simulink model "wind1000_BUS_Original"  (R2024a)
settings: Solver=ode45  StartTime=parSimulacio.ti  StopTime=40  MaxStep=parSimulacio.pas  RelTol=1e-6  AbsTol=1e-6  EnableMultiTasking=on
model workspace (MAT data in the model file):
  tt_vw = Simulink.Parameter
  tt_vw.Complexity = 'real'
  tt_vw.Dimensions = [0 0]
  tt_vw.DataType = 'auto'
values from neighbouring files (symbols this model uses):
  L = L'    [parameters_windFarm2.m]
  nu = size(S, 1)    [parameters_windFarm2.m]
undefined symbols (not defined in the file or in its folder): parControl, parConvertidor, parGenerador, parGrid, parMecanica, parSimulacio, S, w0, windspeed
(part 1 of 218)
settings Model: SimulationMode=accelerator  Creator=adria  LastModifiedBy=hz1021
settings SolverCC: AlgebraicLoopSolver=TrustRegion
settings DataIOCC: LimitDataPoints=on  SaveFormat=Array  SignalLoggingSaveFormat=ModelDataLogs  SaveOutput=off  SaveTime=off  ReturnWorkspaceOutputs=off
settings OptimizationCC: ReuseModelBlockBuffer=off  InitFltsAndDblsToZero=on  LifeSpan=inf
settings DebuggingCC: SaveWithParameterizedLinksMsg=none  UnderspecifiedInitializationDetection=Classic  MergeDetectMultiDrivingBlocksExec=none  SignalResolutionControl=TryResolveAllWithWarning  SolverPrmCheckMsg=warning  FcnCallInpInsideContextMsg=warning  UnconnectedInputMsg=warning  UnconnectedOutputMsg=warning  UnconnectedLineMsg=warning  SFUnexpectedBacktrackingDiag=warning  SFNoUnconditionalDefaultTransitionDiag=warning  SFExecutionAtInitializationDiag=none  ArithmeticOperatorsInVariantConditions=warning
settings HardwareCC: ProdBitPerPointer=32  ProdBitPerSizeT=32  ProdBitPerPtrDiffT=32  ProdLargestAtomicFloat=None  ProdIntDivRoundTo=Undefined  ProdEndianess=Unspecified  ProdWordSize=32  ProdHWDeviceType=32-bit Generic
settings ModelReferenceCC: EnableRefExpFcnMdlSchedulingChecks=on  PropagateSignalLabelsOutOfModel=off
settings RTWCC: GPUComputeCapability=3.5  DLArmComputeVersion=19.05
settings CodeAppCC: StateflowObjectComments=on
settings GRTTargetCC: TargetFcnLib=ansi_tfl_tmw.mat  TargetLangStandard=C89/C90 (ANSI)  CombineOutputUpdateFcns=off  GRTInterface=on
settings ConfigComp: CovMetricSettings=dw  CovSaveCumulativeToWorkspaceVar=on  CovSaveSingleToWorkspaceVar=on

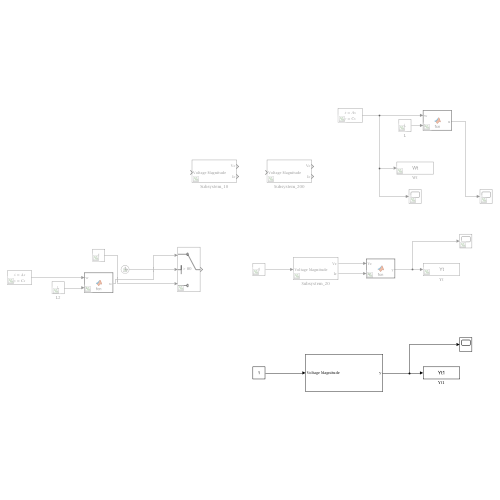
[thumbnail: Simulink's stored preview of the top-level diagram]
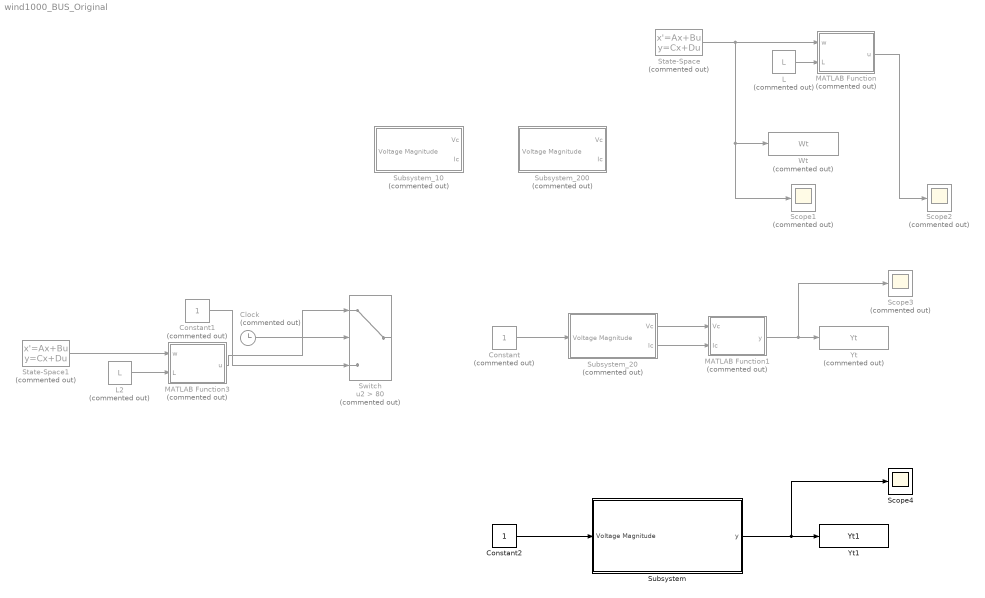
== system wind1000_BUS_Original ==
  Clock : Clock  Commented=on
  Constant : Constant  Commented=on
  Constant1 : Constant  Commented=on
  Constant2 : Constant
  L : Constant  Commented=on  Value=L
  L2 : Constant  Commented=on  Value=L
  MATLAB Function : MATLAB Function  Commented=on  TreatAsAtomicUnit=on
  MATLAB Function1 : MATLAB Function  Commented=on  TreatAsAtomicUnit=on
  MATLAB Function3 : MATLAB Function  Commented=on  TreatAsAtomicUnit=on
  Scope1 : Scope  Commented=on
  Scope2 : Scope  Commented=on
  Scope3 : Scope  Commented=on
  Scope4 : Scope
  State-Space : StateSpace  Commented=on  A=S  B=[]  C=eye(nu)  D=[]  InitialCondition=w0  AbsoluteTolerance=1e-6
  State-Space1 : StateSpace  Commented=on  A=S  B=[]  C=eye(nu)  D=[]  InitialCondition=w0  AbsoluteTolerance=1e-9
  Subsystem : Subsystem
  Subsystem_10 : Subsystem  Commented=on
  Subsystem_20 : Subsystem  Commented=on
  Subsystem_200 : Subsystem  Commented=on
  Switch : Switch  Commented=on  Criteria=u2 > Threshold  Threshold=80  SaturateOnIntegerOverflow=off  InputSameDT=off
  Wt : ToWorkspace  Commented=on  VariableName=Wt  SaveFormat=Timeseries  SampleTime=-1
  Yt : ToWorkspace  Commented=on  VariableName=Yt  SaveFormat=Timeseries  SampleTime=-1
  Yt1 : ToWorkspace  VariableName=Yt1  SaveFormat=Timeseries  SampleTime=-1
  wires:
  State-Space/1 -> Wt/1, Scope1/1, MATLAB Function/1(w)
  MATLAB Function1/1(y) -> Scope3/1, Yt/1
  MATLAB Function/1(u) -> Scope2/1
  L/1 -> MATLAB Function/2(L)
  Subsystem_20/1(Vc) -> MATLAB Function1/1(Vc)
  Subsystem_20/2(Ic) -> MATLAB Function1/2(Ic)
  Constant1/1 -> Switch/3
  L2/1 -> MATLAB Function3/2(L)
  Clock/1 -> Switch/2
  MATLAB Function3/1(u) -> Switch/1
  State-Space1/1 -> MATLAB Function3/1(w)
  Constant/1 -> Subsystem_20/1(Voltage Magnitude)
  Subsystem/1(y) -> Yt1/1, Scope4/1
  Constant2/1 -> Subsystem/1(Voltage Magnitude)

== system wind1000_BUS_Original/Subsystem ==
  Voltage Magnitude : Inport
  MATLAB Function2 : MATLAB Function  TreatAsAtomicUnit=on
  Subsystem_1 : Subsystem
  y : Outport
  wires:
  Subsystem_1/2(Ic) -> MATLAB Function2/2(Ic)
  Voltage Magnitude/1 -> Subsystem_1/1(Voltage Magnitude)
  Subsystem_1/1(Vc) -> MATLAB Function2/1(Vc)
  MATLAB Function2/1(y) -> y/1

== system wind1000_BUS_Original/Subsystem_10 ==
  Voltage Magnitude : Inport
  3phase2dq : Subsystem
  C at HV transf : Subsystem
  Gain1 : Gain  Gain=1/parGrid.WFtransf.rloss
  HV transf1 : Subsystem
  String1 : Subsystem
  Subtract1 : Sum  Inputs=+-
  Vc : Outport
  Ic : Outport  Port=2
  wires:
  Subtract1/1 -> C at HV transf/2(Itransf)
  3phase2dq/1(Vdq) -> HV transf1/1(Vin)
  HV transf1/1(Itransf) -> Subtract1/1
  C at HV transf/1(Vc) -> Vc/1, String1/1(V_in), HV transf1/2(Vc), Gain1/1
  Gain1/1 -> Subtract1/2
  Voltage Magnitude/1 -> 3phase2dq/1(Voltage Magnitude)
  String1/1(I_out) -> Ic/1, C at HV transf/1(Istrings)

== system wind1000_BUS_Original/Subsystem_20 ==
  Voltage Magnitude : Inport
  3phase2dq : Subsystem
  C at HV transf : Subsystem
  Gain1 : Gain  Gain=1/parGrid.WFtransf.rloss
  HV transf1 : Subsystem
  Scope1 : Scope
  Scope3 : Scope
  Subsystem : Subsystem
  Subtract1 : Sum  Inputs=+-
  Vc : Outport
  Ic : Outport  Port=2
  wires:
  Subtract1/1 -> Scope3/1, Ic/1, C at HV transf/2(Itransf)
  3phase2dq/1(Vdq) -> HV transf1/1(Vin)
  HV transf1/1(Itransf) -> Subtract1/1
  C at HV transf/1(Vc) -> Subsystem/1(V_in), Scope1/1, Vc/1, HV transf1/2(Vc), Gain1/1
  Gain1/1 -> Subtract1/2
  Voltage Magnitude/1 -> 3phase2dq/1(Voltage Magnitude)
  Subsystem/1(I_out_tol) -> C at HV transf/1(Istrings)

== system wind1000_BUS_Original/Subsystem_200 ==
  Voltage Magnitude : Inport
  3phase2dq : Subsystem
  C at HV transf : Subsystem
  Gain1 : Gain  Gain=1/parGrid.WFtransf.rloss
  HV transf1 : Subsystem
  Scope1 : Scope
  Scope3 : Scope
  Subsystem : Subsystem
  Subtract1 : Sum  Inputs=+-
  Vc : Outport
  Ic : Outport  Port=2
  wires:
  Subtract1/1 -> Scope3/1, Ic/1, C at HV transf/2(Itransf)
  3phase2dq/1(Vdq) -> HV transf1/1(Vin)
  HV transf1/1(Itransf) -> Subtract1/1
  C at HV transf/1(Vc) -> Subsystem/1(V_in), Scope1/1, Vc/1, HV transf1/2(Vc), Gain1/1
  Gain1/1 -> Subtract1/2
  Voltage Magnitude/1 -> 3phase2dq/1(Voltage Magnitude)
  Subsystem/1(I_out_tol) -> C at HV transf/1(Istrings)

== system wind1000_BUS_Original/Subsystem/Subsystem_1 ==
  Voltage Magnitude : Inport
  3phase2dq : Subsystem
  C at HV transf : Subsystem
  Gain1 : Gain  Gain=1/parGrid.WFtransf.rloss
  HV transf1 : Subsystem
  Scope1 : Scope
  Scope3 : Scope
  Subsystem : Subsystem
  Subtract1 : Sum  Inputs=+-
  Vc : Outport
  Ic : Outport  Port=2
  wires:
  Subtract1/1 -> Scope3/1, Ic/1, C at HV transf/2(Itransf)
  3phase2dq/1(Vdq) -> HV transf1/1(Vin)
  HV transf1/1(Itransf) -> Subtract1/1
  C at HV transf/1(Vc) -> Subsystem/1(V_in), Scope1/1, Vc/1, HV transf1/2(Vc), Gain1/1
  Gain1/1 -> Subtract1/2
  Voltage Magnitude/1 -> 3phase2dq/1(Voltage Magnitude)
  Subsystem/1(I_out_tol) -> C at HV transf/1(Istrings)

== system wind1000_BUS_Original/Subsystem_10/3phase2dq ==
  Voltage Magnitude : Inport
  Clock2 : Clock
  Font trifasica asimetrica1 : Subsystem  mask{tempPar=parGrid.WFtransf}
      mask prompts: tempPar "Estruc. Parametres"
      mask init:
      | we=tempPar.f*2*pi;
      | A_D=tempPar.V_D*sqrt(2)/sqrt(3);
      | A_I=tempPar.V_I*sqrt(2)/sqrt(3);
      | phi_D=tempPar.phi_D;
      | phi_I=tempPar.phi_I;
      | tt_D=tempPar.tt_D;
      | tt_I=tempPar.tt_I;
      | tA_D=tempPar.tA_D;
      | tA_I=tempPar.tA_I;
  Product : Product
  Vdq : Outport
  wires:
  Product/1 -> Vdq/1
  Clock2/1 -> Font trifasica asimetrica1/1(t)
  Font trifasica asimetrica1/1(y) -> Product/1
  Voltage Magnitude/1 -> Product/2

== system wind1000_BUS_Original/Subsystem_10/C at HV transf ==
  note: Vc_cable
  Istrings : Inport
  Itransf : Inport  Port=2
  Add : Sum  Inputs=+-
  produce Vc_cable : StateSpace  A=[0,-100*pi;100*pi,0]  B=eye(2)/parGrid.WFtransf.Cfilt  C=eye(2)  D=zeros(2,2)  InitialCondition=sqrt(2)*66000/sqrt(3)*[1;0]
  Vc : Outport
  wires:
  produce Vc_cable/1 -> Vc/1
  Add/1 -> produce Vc_cable/1
  Itransf/1 -> Add/2
  Istrings/1 -> Add/1

== system wind1000_BUS_Original/Subsystem_10/HV transf1 ==
  Vin : Inport
  Vc : Inport  Port=2
  1/L_Cable : Gain  Gain=1/parGrid.WFtransf.L
  A : Constant  Value=[parGrid.WFtransf.r,100*pi*parGrid.WFtransf.L;-100*pi*parGrid.WFtransf.L,parGrid.WFtransf.r]
  Add : Sum  Inputs=+--
  Integrator : Integrator
  Product6 : Product  Multiplication=Matrix(*)
  Itransf : Outport
  wires:
  Integrator/1 -> Product6/2, Itransf/1
  Vc/1 -> Add/1
  Vin/1 -> Add/2
  A/1 -> Product6/1
  Product6/1 -> Add/3
  1/L_Cable/1 -> Integrator/1
  Add/1 -> 1/L_Cable/1

== system wind1000_BUS_Original/Subsystem_10/String1 ==
  V_in : Inport
  Constant : Constant  Value=0
  Scope : Scope
  WT1 : Subsystem
  WT10 : Subsystem
  WT2 : Subsystem
  WT3 : Subsystem
  WT4 : Subsystem
  WT5 : Subsystem
  WT6 : Subsystem
  WT7 : Subsystem
  WT8 : Subsystem
  WT9 : Subsystem
  I_out : Outport
  wires:
  WT10/1(V_out) -> Scope/1
  WT7/1(V_out) -> WT8/1(V_in)
  Constant/1 -> WT10/2(I_in)
  WT4/1(V_out) -> WT5/1(V_in)
  WT4/2(I_out) -> WT3/2(I_in)
  WT3/1(V_out) -> WT4/1(V_in)
  WT1/1(V_out) -> WT2/1(V_in)
  WT10/2(I_out) -> WT9/2(I_in)
  WT5/1(V_out) -> WT6/1(V_in)
  WT2/1(V_out) -> WT3/1(V_in)
  WT7/2(I_out) -> WT6/2(I_in)
  WT8/1(V_out) -> WT9/1(V_in)
  WT6/1(V_out) -> WT7/1(V_in)
  WT9/2(I_out) -> WT8/2(I_in)
  WT5/2(I_out) -> WT4/2(I_in)
  WT2/2(I_out) -> WT1/2(I_in)
  V_in/1 -> WT1/1(V_in)
  WT3/2(I_out) -> WT2/2(I_in)
  WT8/2(I_out) -> WT7/2(I_in)
  WT9/1(V_out) -> WT10/1(V_in)
  WT6/2(I_out) -> WT5/2(I_in)
  WT1/2(I_out) -> I_out/1

== system wind1000_BUS_Original/Subsystem_20/3phase2dq ==
  Voltage Magnitude : Inport
  Clock2 : Clock
  Font trifasica asimetrica1 : Subsystem  mask{tempPar=parGrid.WFtransf}
      mask prompts: tempPar "Estruc. Parametres"
      mask init: same as wind1000_BUS_Original/Subsystem_10/3phase2dq/Font trifasica asimetrica1
  Product : Product
  Vdq : Outport
  wires:
  Product/1 -> Vdq/1
  Clock2/1 -> Font trifasica asimetrica1/1(t)
  Font trifasica asimetrica1/1(y) -> Product/1
  Voltage Magnitude/1 -> Product/2

== system wind1000_BUS_Original/Subsystem_20/C at HV transf ==
  note: Vc_cable
  Istrings : Inport
  Itransf : Inport  Port=2
  Add : Sum  Inputs=+-
  produce Vc_cable : StateSpace  A=[0,-100*pi;100*pi,0]  B=eye(2)/parGrid.WFtransf.Cfilt  C=eye(2)  D=zeros(2,2)  InitialCondition=sqrt(2)*66000/sqrt(3)*[1;0]
  Vc : Outport
  wires:
  produce Vc_cable/1 -> Vc/1
  Add/1 -> produce Vc_cable/1
  Itransf/1 -> Add/2
  Istrings/1 -> Add/1

== system wind1000_BUS_Original/Subsystem_20/HV transf1 ==
  Vin : Inport
  Vc : Inport  Port=2
  1/L_Cable : Gain  Gain=1/parGrid.WFtransf.L
  A : Constant  Value=[parGrid.WFtransf.r,100*pi*parGrid.WFtransf.L;-100*pi*parGrid.WFtransf.L,parGrid.WFtransf.r]
  Add : Sum  Inputs=+--
  Integrator : Integrator
  Product6 : Product  Multiplication=Matrix(*)
  Itransf : Outport
  wires:
  Integrator/1 -> Product6/2, Itransf/1
  Vc/1 -> Add/1
  Vin/1 -> Add/2
  A/1 -> Product6/1
  Product6/1 -> Add/3
  1/L_Cable/1 -> Integrator/1
  Add/1 -> 1/L_Cable/1

== system wind1000_BUS_Original/Subsystem_20/Subsystem ==
  V_in : Inport
  Add : Sum  Inputs=6
  String1 : Subsystem
  String2 : Subsystem
  String3 : Subsystem
  String4 : Subsystem
  String5 : Subsystem
  String6 : Subsystem
  I_out_tol : Outport
  wires:
  V_in/1 -> String6/1(V_in), String5/1(V_in), String4/1(V_in), String3/1(V_in), String2/1(V_in), String1/1(V_in)
  String1/1(I_out) -> Add/1
  String2/1(I_out) -> Add/2
  Add/1 -> I_out_tol/1
  String3/1(I_out) -> Add/3
  String4/1(I_out) -> Add/4
  String5/1(I_out) -> Add/5
  String6/1(I_out) -> Add/6

== system wind1000_BUS_Original/Subsystem_200/3phase2dq ==
  Voltage Magnitude : Inport
  Clock2 : Clock
  Font trifasica asimetrica1 : Subsystem  mask{tempPar=parGrid.WFtransf}
      mask prompts: tempPar "Estruc. Parametres"
      mask init: same as wind1000_BUS_Original/Subsystem_10/3phase2dq/Font trifasica asimetrica1
  Product : Product
  Vdq : Outport
  wires:
  Product/1 -> Vdq/1
  Clock2/1 -> Font trifasica asimetrica1/1(t)
  Font trifasica asimetrica1/1(y) -> Product/1
  Voltage Magnitude/1 -> Product/2

== system wind1000_BUS_Original/Subsystem_200/C at HV transf ==
  note: Vc_cable
  Istrings : Inport
  Itransf : Inport  Port=2
  Add : Sum  Inputs=+-
  produce Vc_cable : StateSpace  A=[0,-100*pi;100*pi,0]  B=eye(2)/parGrid.WFtransf.Cfilt  C=eye(2)  D=zeros(2,2)  InitialCondition=sqrt(2)*66000/sqrt(3)*[1;0]
  Vc : Outport
  wires:
  produce Vc_cable/1 -> Vc/1
  Add/1 -> produce Vc_cable/1
  Itransf/1 -> Add/2
  Istrings/1 -> Add/1

== system wind1000_BUS_Original/Subsystem_200/HV transf1 ==
  Vin : Inport
  Vc : Inport  Port=2
  1/L_Cable : Gain  Gain=1/parGrid.WFtransf.L
  A : Constant  Value=[parGrid.WFtransf.r,100*pi*parGrid.WFtransf.L;-100*pi*parGrid.WFtransf.L,parGrid.WFtransf.r]
  Add : Sum  Inputs=+--
  Integrator : Integrator
  Product6 : Product  Multiplication=Matrix(*)
  Itransf : Outport
  wires:
  Integrator/1 -> Product6/2, Itransf/1
  Vc/1 -> Add/1
  Vin/1 -> Add/2
  A/1 -> Product6/1
  Product6/1 -> Add/3
  1/L_Cable/1 -> Integrator/1
  Add/1 -> 1/L_Cable/1

== system wind1000_BUS_Original/Subsystem_200/Subsystem ==
  V_in : Inport
  Add : Sum  Inputs=20
  String1 : Subsystem
  String10 : Subsystem
  String11 : Subsystem
  String12 : Subsystem
  String13 : Subsystem
  String14 : Subsystem
  String15 : Subsystem
  String16 : Subsystem
  String17 : Subsystem
  String18 : Subsystem
  String19 : Subsystem
  String2 : Subsystem
  String20 : Subsystem
  String3 : Subsystem
  String4 : Subsystem
  String5 : Subsystem
  String6 : Subsystem
  String7 : Subsystem
  String8 : Subsystem
  String9 : Subsystem
  I_out_tol : Outport
  wires:
  V_in/1 -> String2/1(V_in), String3/1(V_in), String4/1(V_in), String5/1(V_in), String6/1(V_in), String7/1(V_in), String8/1(V_in), String9/1(V_in), String10/1(V_in), String11/1(V_in), String12/1(V_in), String13/1(V_in), String14/1(V_in), String15/1(V_in), String16/1(V_in), String17/1(V_in), String18/1(V_in), String20/1(V_in), String19/1(V_in), String1/1(V_in)
  String1/1(I_out) -> Add/1
  String17/1(I_out) -> Add/17
  String14/1(I_out) -> Add/14
  String3/1(I_out) -> Add/3
  String5/1(I_out) -> Add/5
  String13/1(I_out) -> Add/13
  String16/1(I_out) -> Add/16
  String9/1(I_out) -> Add/9
  String7/1(I_out) -> Add/7
  String2/1(I_out) -> Add/2
  String12/1(I_out) -> Add/12
  String11/1(I_out) -> Add/11
  String10/1(I_out) -> Add/10
  String20/1(I_out) -> Add/20
  String8/1(I_out) -> Add/8
  String19/1(I_out) -> Add/19
  String15/1(I_out) -> Add/15
  String18/1(I_out) -> Add/18
  String6/1(I_out) -> Add/6
  String4/1(I_out) -> Add/4
  Add/1 -> I_out_tol/1

== system wind1000_BUS_Original/Subsystem/Subsystem_1/3phase2dq ==
  Voltage Magnitude : Inport
  Clock2 : Clock
  Font trifasica asimetrica1 : Subsystem  mask{tempPar=parGrid.WFtransf}
      mask prompts: tempPar "Estruc. Parametres"
      mask init: same as wind1000_BUS_Original/Subsystem_10/3phase2dq/Font trifasica asimetrica1
  Product : Product
  Vdq : Outport
  wires:
  Product/1 -> Vdq/1
  Clock2/1 -> Font trifasica asimetrica1/1(t)
  Font trifasica asimetrica1/1(y) -> Product/1
  Voltage Magnitude/1 -> Product/2

== system wind1000_BUS_Original/Subsystem/Subsystem_1/C at HV transf ==
  note: Vc_cable
  Istrings : Inport
  Itransf : Inport  Port=2
  Add : Sum  Inputs=+-
  produce Vc_cable : StateSpace  A=[0,-100*pi;100*pi,0]  B=eye(2)/parGrid.WFtransf.Cfilt  C=eye(2)  D=zeros(2,2)  InitialCondition=sqrt(2)*66000/sqrt(3)*[1;0]
  Vc : Outport
  wires:
  produce Vc_cable/1 -> Vc/1
  Add/1 -> produce Vc_cable/1
  Itransf/1 -> Add/2
  Istrings/1 -> Add/1

== system wind1000_BUS_Original/Subsystem/Subsystem_1/HV transf1 ==
  Vin : Inport
  Vc : Inport  Port=2
  1/L_Cable : Gain  Gain=1/parGrid.WFtransf.L
  A : Constant  Value=[parGrid.WFtransf.r,100*pi*parGrid.WFtransf.L;-100*pi*parGrid.WFtransf.L,parGrid.WFtransf.r]
  Add : Sum  Inputs=+--
  Integrator : Integrator
  Product6 : Product  Multiplication=Matrix(*)
  Itransf : Outport
  wires:
  Integrator/1 -> Product6/2, Itransf/1
  Vc/1 -> Add/1
  Vin/1 -> Add/2
  A/1 -> Product6/1
  Product6/1 -> Add/3
  1/L_Cable/1 -> Integrator/1
  Add/1 -> 1/L_Cable/1

== system wind1000_BUS_Original/Subsystem/Subsystem_1/Subsystem ==
  V_in : Inport
  Add : Sum  Inputs=6
  String1 : Subsystem
  String2 : Subsystem
  String3 : Subsystem  Commented=on
  String4 : Subsystem  Commented=on
  String5 : Subsystem  Commented=on
  String6 : Subsystem  Commented=on
  I_out_tol : Outport
  wires:
  V_in/1 -> String6/1(V_in), String5/1(V_in), String4/1(V_in), String3/1(V_in), String2/1(V_in), String1/1(V_in)
  String1/1(I_out) -> Add/1
  String2/1(I_out) -> Add/2
  Add/1 -> I_out_tol/1
  String3/1(I_out) -> Add/3
  String4/1(I_out) -> Add/4
  String5/1(I_out) -> Add/5
  String6/1(I_out) -> Add/6

== system wind1000_BUS_Original/Subsystem_10/3phase2dq/Font trifasica asimetrica1 ==
  t : Inport
  Clock : Clock
  Constant1 : Constant  Value=phi_D
  Constant2 : Constant  Value=phi_I
  Constant3 : Constant  Value=2*pi/3
  Constant4 : Constant  Value=2*pi/3
  Gain : Gain  Gain=we
  Gain1 : Gain  Gain=A_D
  Gain2 : Gain  Gain=A_I
  Mux : Mux  Inputs=3
  Mux1 : Mux  Inputs=3
  Park : Subsystem
  Product : Product  RndMeth=Zero
  Product1 : Product  RndMeth=Zero
  Product4 : Product  Multiplication=Matrix(*)  RndMeth=Zero
  Scope1 : Scope
  Sum{1,3,5..7} (5 alike) : Sum  Inputs=|++
  Sum2 : Sum  Inputs=|+-
  Sum4 : Sum  Inputs=|+-
  Trigonometric Function{1..6} (6 alike) : Trigonometry  Operator=cos
  directa : Lookup  InputValues=tt_D  Table=tA_D  SaturateOnIntegerOverflow=off
  inversa : Lookup  InputValues=tt_I  Table=tA_I  SaturateOnIntegerOverflow=off
  we : Gain  Gain=100*pi
  y : Outport
  wires:
  Sum3/1 -> Trigonometric Function2/1, Sum2/1, Sum1/1
  Sum2/1 -> Trigonometric Function1/1
  Sum1/1 -> Trigonometric Function3/1
  Constant4/1 -> Sum2/2, Sum1/2
  Trigonometric Function2/1 -> Mux/1
  Trigonometric Function1/1 -> Mux/2
  Trigonometric Function3/1 -> Mux/3
  Mux/1 -> Gain1/1
  t/1 -> Gain/1, directa/1, inversa/1
  Gain/1 -> Sum3/1, Sum6/1
  Constant1/1 -> Sum3/2
  Sum6/1 -> Trigonometric Function5/1, Sum5/1, Sum4/1
  Sum5/1 -> Trigonometric Function4/1
  Sum4/1 -> Trigonometric Function6/1
  Constant3/1 -> Sum4/2, Sum5/2
  Trigonometric Function5/1 -> Mux1/1
  Trigonometric Function4/1 -> Mux1/2
  Trigonometric Function6/1 -> Mux1/3
  Mux1/1 -> Gain2/1
  Constant2/1 -> Sum6/2
  Product/1 -> Sum7/1
  directa/1 -> Product/1
  inversa/1 -> Product1/1
  Gain1/1 -> Product/2
  Gain2/1 -> Product1/2
  Product1/1 -> Sum7/2
  Park/1(Tthetar) -> Scope1/1, Product4/1
  Clock/1 -> we/1
  we/1 -> Park/1(theta_r)
  Sum7/1 -> Product4/2
  Product4/1 -> y/1

== system wind1000_BUS_Original/Subsystem_10/String1/WT1 ==
  V_in : Inport
  I_in : Inport  Port=2
  Add1 : Sum  Inputs=+-
  C_cable : Subsystem
  C_filter : Subsystem
  Cable line : Subsystem
  Gain : Gain  Gain=1/parGrid.WTstring.Rparallel_cable
  Transformer-complex : Subsystem
  WFG : Subsystem
  V_out : Outport
  I_out : Outport  Port=2
  wires:
  C_cable/1(Vc_cable) -> Gain/1, Transformer-complex/2(Vc_cable_qd), V_out/1, Cable line/2(Vc_cable)
  WFG/1(ilqd) -> C_filter/1(IWF)
  C_filter/1(Vc_filter_qd) -> Transformer-complex/1(Vc_filter_qd), WFG/1(vcqd)
  Cable line/1(I_cable) -> I_out/1, C_cable/3(I_cable)
  Transformer-complex/1(It1) -> C_filter/2(It1)
  V_in/1 -> Cable line/1(V)
  Transformer-complex/2(It2) -> Add1/1
  Add1/1 -> C_cable/1(It2)
  Gain/1 -> Add1/2
  I_in/1 -> C_cable/2(I_in)

== system wind1000_BUS_Original/Subsystem_10/String1/WT10 ==
  V_in : Inport
  I_in : Inport  Port=2
  Add1 : Sum  Inputs=+-
  C_cable : Subsystem
  C_filter : Subsystem
  Cable line : Subsystem
  Gain : Gain  Gain=1/parGrid.WTstring.Rparallel_cable
  Transformer-complex : Subsystem
  WFG : Subsystem
  V_out : Outport
  I_out : Outport  Port=2
  wires:
  I_in/1 -> C_cable/2(I_in)
  Gain/1 -> Add1/2
  Add1/1 -> C_cable/1(It2)
  Transformer-complex/2(It2) -> Add1/1
  V_in/1 -> Cable line/1(V)
  Transformer-complex/1(It1) -> C_filter/2(It1)
  Cable line/1(I_cable) -> C_cable/3(I_cable), I_out/1
  C_filter/1(Vc_filter_qd) -> WFG/1(vcqd), Transformer-complex/1(Vc_filter_qd)
  WFG/1(ilqd) -> C_filter/1(IWF)
  C_cable/1(Vc_cable) -> Cable line/2(Vc_cable), V_out/1, Transformer-complex/2(Vc_cable_qd), Gain/1

== system wind1000_BUS_Original/Subsystem_10/String1/WT2 ==
  V_in : Inport
  I_in : Inport  Port=2
  Add1 : Sum  Inputs=+-
  C_cable : Subsystem
  C_filter : Subsystem
  Cable line : Subsystem
  Gain : Gain  Gain=1/parGrid.WTstring.Rparallel_cable
  Transformer-complex : Subsystem
  WFG : Subsystem
  V_out : Outport
  I_out : Outport  Port=2
  wires:
  I_in/1 -> C_cable/2(I_in)
  Gain/1 -> Add1/2
  Add1/1 -> C_cable/1(It2)
  Transformer-complex/2(It2) -> Add1/1
  V_in/1 -> Cable line/1(V)
  Transformer-complex/1(It1) -> C_filter/2(It1)
  Cable line/1(I_cable) -> C_cable/3(I_cable), I_out/1
  C_filter/1(Vc_filter_qd) -> WFG/1(vcqd), Transformer-complex/1(Vc_filter_qd)
  WFG/1(ilqd) -> C_filter/1(IWF)
  C_cable/1(Vc_cable) -> Cable line/2(Vc_cable), V_out/1, Transformer-complex/2(Vc_cable_qd), Gain/1

== system wind1000_BUS_Original/Subsystem_10/String1/WT3 ==
  V_in : Inport
  I_in : Inport  Port=2
  Add1 : Sum  Inputs=+-
  C_cable : Subsystem
  C_filter : Subsystem
  Cable line : Subsystem
  Gain : Gain  Gain=1/parGrid.WTstring.Rparallel_cable
  Transformer-complex : Subsystem
  WFG : Subsystem
  V_out : Outport
  I_out : Outport  Port=2
  wires:
  C_cable/1(Vc_cable) -> Gain/1, Transformer-complex/2(Vc_cable_qd), V_out/1, Cable line/2(Vc_cable)
  WFG/1(ilqd) -> C_filter/1(IWF)
  C_filter/1(Vc_filter_qd) -> WFG/1(vcqd), Transformer-complex/1(Vc_filter_qd)
  Cable line/1(I_cable) -> I_out/1, C_cable/3(I_cable)
  Transformer-complex/1(It1) -> C_filter/2(It1)
  V_in/1 -> Cable line/1(V)
  Transformer-complex/2(It2) -> Add1/1
  Add1/1 -> C_cable/1(It2)
  Gain/1 -> Add1/2
  I_in/1 -> C_cable/2(I_in)

== system wind1000_BUS_Original/Subsystem_10/String1/WT4 ==
  V_in : Inport
  I_in : Inport  Port=2
  Add1 : Sum  Inputs=+-
  C_cable : Subsystem
  C_filter : Subsystem
  Cable line : Subsystem
  Gain : Gain  Gain=1/parGrid.WTstring.Rparallel_cable
  Transformer-complex : Subsystem
  WFG : Subsystem
  V_out : Outport
  I_out : Outport  Port=2
  wires:
  I_in/1 -> C_cable/2(I_in)
  Gain/1 -> Add1/2
  Add1/1 -> C_cable/1(It2)
  Transformer-complex/2(It2) -> Add1/1
  V_in/1 -> Cable line/1(V)
  Transformer-complex/1(It1) -> C_filter/2(It1)
  Cable line/1(I_cable) -> C_cable/3(I_cable), I_out/1
  C_filter/1(Vc_filter_qd) -> WFG/1(vcqd), Transformer-complex/1(Vc_filter_qd)
  WFG/1(ilqd) -> C_filter/1(IWF)
  C_cable/1(Vc_cable) -> Cable line/2(Vc_cable), V_out/1, Transformer-complex/2(Vc_cable_qd), Gain/1

== system wind1000_BUS_Original/Subsystem_10/String1/WT5 ==
  V_in : Inport
  I_in : Inport  Port=2
  Add1 : Sum  Inputs=+-
  C_cable : Subsystem
  C_filter : Subsystem
  Cable line : Subsystem
  Gain : Gain  Gain=1/parGrid.WTstring.Rparallel_cable
  Transformer-complex : Subsystem
  WFG : Subsystem
  V_out : Outport
  I_out : Outport  Port=2
  wires:
  C_cable/1(Vc_cable) -> Gain/1, Transformer-complex/2(Vc_cable_qd), V_out/1, Cable line/2(Vc_cable)
  WFG/1(ilqd) -> C_filter/1(IWF)
  C_filter/1(Vc_filter_qd) -> WFG/1(vcqd), Transformer-complex/1(Vc_filter_qd)
  Cable line/1(I_cable) -> I_out/1, C_cable/3(I_cable)
  Transformer-complex/1(It1) -> C_filter/2(It1)
  V_in/1 -> Cable line/1(V)
  Transformer-complex/2(It2) -> Add1/1
  Add1/1 -> C_cable/1(It2)
  Gain/1 -> Add1/2
  I_in/1 -> C_cable/2(I_in)
